simulink model "CCMSysServ"  (R2022b) (continued)
== system CCMSysServ == (continued)
      | <ReqID>NULL</ReqID>
      | <InitValue>0</InitValue>
  CarCfg_CarCfg790 : Inport  Description=|  Port=580  OutDataTypeStr=Nr2  PortDimensions=1
      | Energy adjustable type
      | <to>UsgModMgr</to>
      | <typ>Internal</typ>
      | <ReqID>22092</ReqID>
      | <InitValue>0</InitValue>
  CarCfg_CarCfg793 : Inport  Description=|  Port=581  OutDataTypeStr=Nr2  PortDimensions=1
      | VEHICLE TYPE
      | <to>All</to>
      | <typ>Internal</typ>
      | <ReqID>NULL</ReqID>
      | <InitValue>0</InitValue>
  CarCfg_CarCfg794 : Inport  Description=|  Port=582  OutDataTypeStr=Nr2  PortDimensions=1
      | FOOT SUPPORT
      | <to>All</to>
      | <typ>Internal</typ>
      | <ReqID>198565</ReqID>
      | <InitValue>0</InitValue>
  CarCfg_CarCfg798 : Inport  Description=|  Port=583  OutDataTypeStr=Nr2  PortDimensions=1
      | VEHICLE TYPE
      | <to>All</to>
      | <typ>Internal</typ>
      | <ReqID>NULL</ReqID>
      | <InitValue>0</InitValue>
  CarCfg_CarCfg8 : Inport  Description=|  Port=584  OutDataTypeStr=Nr2  PortDimensions=1
      | STEERING WHEEL POSITION
      | <to>All</to>
      | <typ>Internal</typ>
      | <ReqID>NULL</ReqID>
      | <InitValue>0</InitValue>
  CarCfg_CarCfg80 : Inport  Description=|  Port=585  OutDataTypeStr=Nr2  PortDimensions=1
      | KEYLESS ANTENNAS
      | <to>All</to>
      | <typ>Internal</typ>
      | <ReqID>NULL</ReqID>
      | <InitValue>0</InitValue>
  CarCfg_CarCfg81 : Inport  Description=|  Port=586  OutDataTypeStr=Nr2  PortDimensions=1
      | DOOR HANDLE TYPE
      | <to>All</to>
      | <typ>Internal</typ>
      | <ReqID>NULL</ReqID>
      | <InitValue>0</InitValue>
  CarCfg_CarCfg811 : Inport  Description=|  Port=587  OutDataTypeStr=Nr2  PortDimensions=1
      | VEHICLE TYPE
      | <to>All</to>
      | <typ>Internal</typ>
      | <ReqID>NULL</ReqID>
      | <InitValue>0</InitValue>
  CarCfg_CarCfg814 : Inport  Description=|  Port=588  OutDataTypeStr=Nr2  PortDimensions=1
      | VEHICLE TYPE
      | <to>All</to>
      | <typ>Internal</typ>
      | <ReqID>NULL</ReqID>
      | <InitValue>0</InitValue>
  CarCfg_CarCfg816 : Inport  Description=|  Port=589  OutDataTypeStr=Nr2  PortDimensions=1
      | VEHICLE TYPE
      | <to>All</to>
      | <typ>Internal</typ>
      | <ReqID>NULL</ReqID>
      | <InitValue>0</InitValue>
  CarCfg_CarCfg82 : Inport  Description=|  Port=590  OutDataTypeStr=Nr2  PortDimensions=1
      | CHARGE LID LOCK
      | <to>All</to>
      | <typ>Internal</typ>
      | <ReqID>NULL</ReqID>
      | <InitValue>0</InitValue>
  CarCfg_CarCfg822 : Inport  Description=|  Port=591  OutDataTypeStr=Nr2  PortDimensions=1
      | VEHICLE TYPE
      | <to>All</to>
      | <typ>Internal</typ>
      | <ReqID>NULL</ReqID>
      | <InitValue>0</InitValue>
  CarCfg_CarCfg826 : Inport  Description=|  Port=592  OutDataTypeStr=Nr2  PortDimensions=1
      | 12V Delayed Power Off
      | <to>All</to>
      | <typ>Internal</typ>
      | <ReqID>22010</ReqID>
      | <InitValue>0</InitValue>
  CarCfg_CarCfg829 : Inport  Description=|  Port=593  OutDataTypeStr=Nr2  PortDimensions=1
      | VEHICLE TYPE
      | <to>All</to>
      | <typ>Internal</typ>
      | <ReqID>NULL</ReqID>
      | <InitValue>0</InitValue>
  CarCfg_CarCfg83 : Inport  Description=|  Port=594  OutDataTypeStr=Nr2  PortDimensions=1
      | FUEL LID LOCK
      | <to>All</to>
      | <typ>Internal</typ>
      | <ReqID>NULL</ReqID>
      | <InitValue>0</InitValue>
  CarCfg_CarCfg831 : Inport  Description=|  Port=595  OutDataTypeStr=Nr2  PortDimensions=1
      | VEHICLE TYPE
      | <to>All</to>
      | <typ>Internal</typ>
      | <ReqID>NULL</ReqID>
      | <InitValue>0</InitValue>
  CarCfg_CarCfg834 : Inport  Description=|  Port=596  OutDataTypeStr=Nr2  PortDimensions=1
      | A2 Front position lamp
      | <to>All</to>
      | <typ>Internal</typ>
      | <ReqID>670781</ReqID>
      | <InitValue>0</InitValue>
  CarCfg_CarCfg835 : Inport  Description=|  Port=597  OutDataTypeStr=Nr2  PortDimensions=1
      | ROOF DRIVE TYPE
      | <to>All</to>
      | <typ>Internal</typ>
      | <ReqID>/</ReqID>
      | <InitValue>0</InitValue>
  CarCfg_CarCfg837 : Inport  Description=|  Port=598  OutDataTypeStr=Nr2  PortDimensions=1
      | Mobile phone remote monitoring and call
      | <to>UsgModMgr</to>
      | <typ>Internal</typ>
      | <ReqID>22019</ReqID>
      | <InitValue>0</InitValue>
  CarCfg_CarCfg839 : Inport  Description=|  Port=599  OutDataTypeStr=Nr2  PortDimensions=1
      | Energy adjustable type
      | <to>UsgModMgr</to>
      | <typ>Internal</typ>
      | <ReqID>22092</ReqID>
      | <InitValue>0</InitValue>
  CarCfg_CarCfg84 : Inport  Description=|  Port=600  OutDataTypeStr=Nr2  PortDimensions=1
      | CAR LOCK TYPE
      | <to>All</to>
      | <typ>Internal</typ>
      | <ReqID>NULL</ReqID>
      | <InitValue>0</InitValue>
  CarCfg_CarCfg841 : Inport  Description=|  Port=601  OutDataTypeStr=Nr2  PortDimensions=1
      | Electric lifting module
      | <to>All</to>
      | <typ>Internal</typ>
      | <ReqID>/</ReqID>
      | <InitValue>0</InitValue>
  CarCfg_CarCfg842 : Inport  Description=|  Port=602  OutDataTypeStr=Nr2  PortDimensions=1
      | Cabin oxygen supply system
      | <to>CCM</to>
      | <typ>Internal_other</typ>
      | <ReqID>871504</ReqID>
      | <InitValue>0</InitValue>
  CarCfg_CarCfg846 : Inport  Description=|  Port=603  OutDataTypeStr=Nr2  PortDimensions=1
      | RF KEY
      | <to>All</to>
      | <typ>Internal</typ>
      | <ReqID>/</ReqID>
      | <InitValue>0</InitValue>
  CarCfg_CarCfg848 : Inport  Description=|  Port=604  OutDataTypeStr=Nr2  PortDimensions=1
      | VEHICLE TYPE
      | <to>All</to>
      | <typ>Internal</typ>
      | <ReqID>NULL</ReqID>
      | <InitValue>0</InitValue>
  CarCfg_CarCfg849 : Inport  Port=605  OutDataTypeStr=Nr2  PortDimensions=1
  CarCfg_CarCfg85 : Inport  Description=|  Port=606  OutDataTypeStr=Nr2  PortDimensions=1
      | CENTRAL LOCK TYPE
      | <to>All</to>
      | <typ>Internal</typ>
      | <ReqID>NULL</ReqID>
      | <InitValue>0</InitValue>
  CarCfg_CarCfg851 : Inport  Description=|  Port=607  OutDataTypeStr=Nr2  PortDimensions=1
      | FLOATING DRVING
      | <to>CCM</to>
      | <typ>Internal_other</typ>
      | <ReqID>858088</ReqID>
      | <InitValue>0</InitValue>
  CarCfg_CarCfg855 : Inport  Description=|  Port=608  OutDataTypeStr=Nr2  PortDimensions=1
      | VEHICLE TYPE
      | <to>All</to>
      | <typ>Internal</typ>
      | <ReqID>NULL</ReqID>
      | <InitValue>0</InitValue>
  CarCfg_CarCfg857 : Inport  Description=|  Port=609  OutDataTypeStr=Nr2  PortDimensions=1
      | Ceiling Screen Control
      | <to>ALL</to>
      | <typ>Internal</typ>
      | <ReqID>/</ReqID>
      | <InitValue>0</InitValue>
  CarCfg_CarCfg858 : Inport  Description=|  Port=610  OutDataTypeStr=Nr2  PortDimensions=1
      | HIDDEN REAR WINDOW WIPER
      | <to>WiprWshrMgr</to>
      | <typ>Internal</typ>
      | <ReqID>169786</ReqID>
      | <InitValue>0</InitValue>
  CarCfg_CarCfg86 : Inport  Description=|  Port=611  OutDataTypeStr=Nr2  PortDimensions=1
      | CENTRAL LOCK SWITCHES
      | <to>All</to>
      | <typ>Internal</typ>
      | <ReqID>NULL</ReqID>
      | <InitValue>0</InitValue>
  CarCfg_CarCfg87 : Inport  Description=|  Port=612  OutDataTypeStr=Nr2  PortDimensions=1
      | CHILD LOCK, REAR DOORS
      | <to>All</to>
      | <typ>Internal</typ>
      | <ReqID>NULL</ReqID>
      | <InitValue>0</InitValue>
  CarCfg_CarCfg88 : Inport  Description=|  Port=613  OutDataTypeStr=Nr2  PortDimensions=1
      | PRIVATE LOCKING, LUGGAGE COMP.
      | <to>All</to>
      | <typ>Internal</typ>
      | <ReqID>NULL</ReqID>
      | <InitValue>0</InitValue>
  CarCfg_CarCfg89 : Inport  Description=|  Port=614  OutDataTypeStr=Nr2  PortDimensions=1
      | SLAM LOCKING
      | <to>All</to>
      | <typ>Internal</typ>
      | <ReqID>NULL</ReqID>
      | <InitValue>0</InitValue>
  CarCfg_CarCfg9 : Inport  Description=|  Port=615  OutDataTypeStr=Nr2  PortDimensions=1
      | GEARBOX
      | <to>All</to>
      | <typ>Internal</typ>
      | <ReqID>NULL</ReqID>
      | <InitValue>0</InitValue>
  CarCfg_CarCfg90 : Inport  Description=|  Port=616  OutDataTypeStr=Nr2  PortDimensions=1
      | AUTOMATIC LOCKING BY SPEED
      | <to>All</to>
      | <typ>Internal</typ>
      | <ReqID>NULL</ReqID>
      | <InitValue>0</InitValue>
  CarCfg_CarCfg91 : Inport  Description=|  Port=617  OutDataTypeStr=Nr2  PortDimensions=1
      | TWO STEP UNLOCKING
      | <to>All</to>
      | <typ>Internal</typ>
      | <ReqID>NULL</ReqID>
      | <InitValue>0</InitValue>
  CarCfg_CarCfg92 : Inport  Description=|  Port=618  OutDataTypeStr=Nr2  PortDimensions=1
      | FREQUENCY, REMOTE CONTROLS
      | <to>All</to>
      | <typ>Internal</typ>
      | <ReqID>NULL</ReqID>
      | <InitValue>0</InitValue>
  CarCfg_CarCfg93 : Inport  Description=|  Port=619  OutDataTypeStr=Nr2  PortDimensions=1
      | AUTHENTIC COMMUNICATION OF LOCKING COMMANDS
      | <to>All</to>
      | <typ>Internal</typ>
      | <ReqID>NULL</ReqID>
      | <InitValue>0</InitValue>
  CarCfg_CarCfg94 : Inport  Description=|  Port=620  OutDataTypeStr=Nr2  PortDimensions=1
      | KEYLESS ENTRY
      | <to>All</to>
      | <typ>Internal</typ>
      | <ReqID>NULL</ReqID>
      | <InitValue>0</InitValue>
  CarCfg_CarCfg95 : Inport  Description=|  Port=621  OutDataTypeStr=Nr2  PortDimensions=1
      | VISIBLE LOCKING/UNLOCKING
      | <to>All</to>
      | <typ>Internal</typ>
      | <ReqID>NULL</ReqID>
      | <InitValue>0</InitValue>
  CarCfg_CarCfg96 : Inport  Description=|  Port=622  OutDataTypeStr=Nr2  PortDimensions=1
      | AUDIBLE LOCKING FEEDBACK
      | <to>All</to>
      | <typ>Internal</typ>
      | <ReqID>NULL</ReqID>
      | <InitValue>0</InitValue>
  CarCfg_CarCfg97 : Inport  Description=|  Port=623  OutDataTypeStr=Nr2  PortDimensions=1
      | TRUNK RELEASE, POP-UP
      | <to>All</to>
      | <typ>Internal</typ>
      | <ReqID>NULL</ReqID>
      | <InitValue>0</InitValue>
  CarCfg_CarCfg98 : Inport  Description=|  Port=624  OutDataTypeStr=Nr2  PortDimensions=1
      | SELF OPENING BOOTLID/TAILGATE
      | <to>All</to>
      | <typ>Internal</typ>
      | <ReqID>NULL</ReqID>
      | <InitValue>0</InitValue>
  CarCfg_CarCfg99 : Inport  Description=|  Port=625  OutDataTypeStr=Nr2  PortDimensions=1
      | HANDSFREE OPENER, BOOTLID/TAILGATE
      | <to>All</to>
      | <typ>Internal</typ>
      | <ReqID>NULL</ReqID>
      | <InitValue>0</InitValue>
  VehCfgPrm_VehCfgPrm : Inport  Port=626  OutDataTypeStr=Bus: VehCfgPrm  PortDimensions=1  BusOutputAsStruct=on
  VehCfgPrmExt_VehCfgPrmExt : Inport  Port=627  OutDataTypeStr=Bus: VehCfgPrm  PortDimensions=1  BusOutputAsStruct=on
  VehModMngtGlbSafe1_VehModMngtGlbSafe1 : Inport  Port=628  OutDataTypeStr=Bus: VehModMngtGlbSafe1  PortDimensions=1  BusOutputAsStruct=on
  Vin_Vin : Inport  Port=629  OutDataTypeStr=Bus: Vin1  PortDimensions=1  BusOutputAsStruct=on
  BswLifeCycle_LifeCycle : Inport  Port=630  OutDataTypeStr=Enum: CCMLifeCycleModeGroup  PortDimensions=1
  CCMSysServ_Init : Subsystem
  CCMSysServ_Runnable_sys : Subsystem
  CCMSysServ_VehCfgPrmExt_sys : Subsystem
  CCMSysServ_VehCfgPrm_sys : Subsystem
  CCMSysServ_VehModMngtGlbSafe1_sys : Subsystem
  CCMSysServ_Vin_sys : Subsystem
  NvMBlockCarCfgData : DataStoreMemory  DataStoreName=NvMBlockCarCfgData  InitialValue=NvMBlockCarCfgDataInit  ShowAdditionalParam=off  Dimensions=62  StateMustResolveToSignalObject=on
  NvMBlockDidDataD005 : DataStoreMemory  DataStoreName=NvMBlockDidDataD005  InitialValue=NvMBlockDidDataD005Init  ShowAdditionalParam=off  Dimensions=90  StateMustResolveToSignalObject=on
  NvMBlockDidDataD09A : DataStoreMemory  DataStoreName=NvMBlockDidDataD09A  InitialValue=NvMBlockDidDataD09AInit  ShowAdditionalParam=off  Dimensions=1  StateMustResolveToSignalObject=on
  NvMBlockDidDataE103 : DataStoreMemory  DataStoreName=NvMBlockDidDataE103  InitialValue=NvMBlockDidDataE103Init  ShowAdditionalParam=off  Dimensions=70  StateMustResolveToSignalObject=on
  NvMBlockDidDataF190 : DataStoreMemory  DataStoreName=NvMBlockDidDataF190  InitialValue=NvMBlockDidDataF190Init  ShowAdditionalParam=off  Dimensions=17  StateMustResolveToSignalObject=on
  NvMBlockDidDataF1AB : DataStoreMemory  DataStoreName=NvMBlockDidDataF1AB  InitialValue=NvMBlockDidDataF1ABInit  ShowAdditionalParam=off  Dimensions=8  StateMustResolveToSignalObject=on
  SysServDtcService : Subsystem
  SysServParaService : Subsystem
  CarCfgForClimaCtrl_CarCfgFor3RDROWSEATHEAT : Outport  OutDataTypeStr=UInt8  PortDimensions=1
  CarCfgForClimaCtrl_CarCfgFor3RDROWSEATVENTILATED : Outport  Port=2  OutDataTypeStr=UInt8  PortDimensions=1
  CarCfgForClimaCtrl_CarCfgForBRAND : Outport  Port=3  OutDataTypeStr=UInt8  PortDimensions=1
  CarCfgForClimaCtrl_CarCfgForCabinOxgenSupply : Outport  Port=4  OutDataTypeStr=UInt8  PortDimensions=1
  CarCfgForClimaCtrl_CarCfgForElectricLiftingModule : Outport  Port=5  OutDataTypeStr=UInt8  PortDimensions=1
  CarCfgForClimaCtrl_CarCfgForExtVentnClimaCtrl : Outport  Port=6  OutDataTypeStr=Enum: CarCfgForExtVentnClimaCtrl  PortDimensions=1
  CarCfgForClimaCtrl_CarCfgForTEMPEREDSTORAGEBOX : Outport  Port=7  OutDataTypeStr=UInt8  PortDimensions=1
  CarCfgForClimaCtrl_CarCfgForFloatingDriving : Outport  Port=8  OutDataTypeStr=UInt8  PortDimensions=1
  CarCfgForClimaCtrl_CarCfgForInteriorViewCamera : Outport  Port=9  OutDataTypeStr=Enum: CarCfgForInteriorViewCamera  PortDimensions=1
  CarCfgForClimaCtrl_CarCfgForLifeDetection : Outport  Port=10  OutDataTypeStr=Enum: LifeDetection  PortDimensions=1
  CarCfgForClimaCtrl_CarCfgForMARKET : Outport  Port=11  OutDataTypeStr=UInt8  PortDimensions=1
  CarCfgForClimaCtrl_CarCfgForPowerSeats : Outport  Port=12  OutDataTypeStr=Enum: CarCfgForPowerSeats  PortDimensions=1
  CarCfgForClimaCtrl_CarCfgForPTCInFrontHVAC : Outport  Port=13  OutDataTypeStr=UInt8  PortDimensions=1
  CarCfgForClimaCtrl_CarCfgForRFKEY : Outport  Port=14  OutDataTypeStr=UInt8  PortDimensions=1
  CarCfgForClimaCtrl_CarCfgForVentLiftMotorExiSts : Outport  Port=15  OutDataTypeStr=UInt32  PortDimensions=1
  CarCfgForClimaCtrl_CarCfgForWadingAssistSystem : Outport  Port=16  OutDataTypeStr=UInt8  PortDimensions=1
  CarCfgForClimaCtrl_CarCfgWindFollowHumanMov : Outport  Port=17  OutDataTypeStr=UInt8  PortDimensions=1
  HvacCfgForClimaCtrl_HvacCfgForAQSTypes : Outport  Port=18  OutDataTypeStr=UInt8  PortDimensions=1
  HvacCfgForClimaCtrl_HvacCfgForHvacLowSideRETXVESts : Outport  Port=19  OutDataTypeStr=UInt8  PortDimensions=1
  HvacCfgForClimaCtrl_HvacCfgForHvacReBlowerType : Outport  Port=20  OutDataTypeStr=UInt8  PortDimensions=1
  HvacCfgForClimaCtrl_HvacCfgForHvacReVentPstn : Outport  Port=21  OutDataTypeStr=UInt8  PortDimensions=1
  HvacCfgForClimaCtrl_HvacCfgForHvacSnsrRCmptmtESts : Outport  Port=22  OutDataTypeStr=UInt8  PortDimensions=1
  HvacCfgForClimaCtrl_HvacCfgForHvacSnsrReHexESts : Outport  Port=23  OutDataTypeStr=UInt8  PortDimensions=1
  HvacCfgReqVersionReplace_HvacCfgReqVersionReplace : Outport  Port=24  OutDataTypeStr=UInt8  PortDimensions=1
  SysServCarCfgSts_SysServCCPLin2AQSSts : Outport  Port=25  OutDataTypeStr=Boolean  PortDimensions=1
  SysServCarCfgSts_SysServDUBlowerPwmSignTypeSts : Outport  Port=26  OutDataTypeStr=UInt8  PortDimensions=1
  SysServCarCfgSts_SysServDULin4HFAFStsH : Outport  Port=27  OutDataTypeStr=UInt32  PortDimensions=1
  SysServCarCfgSts_SysServDUReBlowerPwmSignSts : Outport  Port=28  OutDataTypeStr=UInt8  PortDimensions=1
  SysServCarCfgSts_SysServDUReBlowerPwmSts : Outport  Port=29  OutDataTypeStr=UInt8  PortDimensions=1
  SysServCarCfgSts_SysServFloatingMotorSts : Outport  Port=30  OutDataTypeStr=UInt8  PortDimensions=1
  SysServCarCfgSts_SysServFrntHEXSnsrPosn : Outport  Port=31  OutDataTypeStr=UInt8  PortDimensions=1
  SysServCarCfgSts_SysServLowSideRETXVSts : Outport  Port=32  OutDataTypeStr=UInt8  PortDimensions=1
  SysServCarCfgSts_SysServReHEXSnsrPosn : Outport  Port=33  OutDataTypeStr=UInt8  PortDimensions=1
  SysServCarCfgSts_SysServRHFASts : Outport  Port=34  OutDataTypeStr=UInt8  PortDimensions=1
  SysServCarCfgSts_SysServSnsrForFCmptmtTType : Outport  Port=35  OutDataTypeStr=UInt8  PortDimensions=1
  CarCfgForClimaCtrl_CarCfgForAutDemst : Outport  Port=36  OutDataTypeStr=UInt8  PortDimensions=1
  CarCfgForClimaCtrl_CarCfgForAirClng : Outport  Port=37  OutDataTypeStr=Enum: CarCfgForAirClng  PortDimensions=1
  CarCfgForClimaCtrl_CarCfgForAirClnrAdv : Outport  Port=38  OutDataTypeStr=UInt8  PortDimensions=1
  CarCfgForClimaCtrl_CarCfgForAirFrag : Outport  Port=39  OutDataTypeStr=Enum: CarCfgForAirFrag  PortDimensions=1
  CarCfgForClimaCtrl_CarCfgForAirMtrlSnsr : Outport  Port=40  OutDataTypeStr=Enum: CarCfgForAirMtrlSnsrGly  PortDimensions=1
  CarCfgForClimaCtrl_CarCfgForAirQly : Outport  Port=41  OutDataTypeStr=Enum: CarCfgForAirQlyGly  PortDimensions=1
  CarCfgForClimaCtrl_CarCfgForAmntOfSeat : Outport  Port=42  OutDataTypeStr=Enum: CarCfgForAmntOfSeatTyp3  PortDimensions=1
  CarCfgForClimaCtrl_CarCfgForAuxWtrPmp : Outport  Port=43  OutDataTypeStr=Enum: CarCfgForAuxWtrPmp  PortDimensions=1
  CarCfgForClimaCtrl_CarCfgForBypVlvInHvac : Outport  Port=44  OutDataTypeStr=Enum: CarCfgForBypVlvInHvac2  PortDimensions=1
  CarCfgForClimaCtrl_CarCfgForClsVlvInHvac : Outport  Port=45  OutDataTypeStr=Enum: CarCfgForClsVlvInHvac  PortDimensions=1
  CarCfgForClimaCtrl_CarCfgForCmprAcTyp : Outport  Port=46  OutDataTypeStr=UInt8  PortDimensions=1
  CarCfgForClimaCtrl_CarCfgForCmptmtClng : Outport  Port=47  OutDataTypeStr=Enum: CarCfgForCmptmtClng  PortDimensions=1
  CarCfgForClimaCtrl_CarCfgForElecVentAdjmt : Outport  Port=48  OutDataTypeStr=Enum: CarCfgForElecVentAdjmt  PortDimensions=1
  CarCfgForClimaCtrl_CarCfgForFlcTyp : Outport  Port=49  OutDataTypeStr=Enum: CarCfgForFlcTyp  PortDimensions=1
  CarCfgForClimaCtrl_CarCfgForFoldCSD : Outport  Port=50  OutDataTypeStr=Enum: CarCfgForFoldCSD  PortDimensions=1
  CarCfgForClimaCtrl_CarCfgForFuTyp : Outport  Port=51  OutDataTypeStr=Enum: CarCfgForFuTyp  PortDimensions=1
  CarCfgForClimaCtrl_CarCfgForGlassTyp : Outport  Port=52  OutDataTypeStr=Enum: CarCfgForGlassTyp  PortDimensions=1
  CarCfgForClimaCtrl_CarCfgForHeadRadar : Outport  Port=53  OutDataTypeStr=Enum: HeadRadar  PortDimensions=1
  CarCfgForClimaCtrl_CarCfgForHeatPmp : Outport  Port=54  OutDataTypeStr=Enum: CarCfgForHeatPmpTyp  PortDimensions=1
  CarCfgForClimaCtrl_CarCfgForHeatrDurgDrvg : Outport  Port=55  OutDataTypeStr=Enum: CarCfgForHeatrDurgDrvg  PortDimensions=1
  CarCfgForClimaCtrl_CarCfgForHeatrTyp : Outport  Port=56  OutDataTypeStr=UInt8  PortDimensions=1
  CarCfgForClimaCtrl_CarCfgForHvacFilTyp : Outport  Port=57  OutDataTypeStr=Enum: CarCfgForHvacFilTypGly  PortDimensions=1
  CarCfgForClimaCtrl_CarCfgForHvacFlrSnsrForT : Outport  Port=58  OutDataTypeStr=Enum: CarCfgForHvacFlrSnsrForT  PortDimensions=1
  CarCfgForClimaCtrl_CarCfgForHvacHeatrSnsrT : Outport  Port=59  OutDataTypeStr=Enum: CarCfgForHvacHeatrSnsrT  PortDimensions=1
  CarCfgForClimaCtrl_CarCfgForHvacRe : Outport  Port=60  OutDataTypeStr=UInt8  PortDimensions=1
  CarCfgForClimaCtrl_CarCfgForHvacTyp : Outport  Port=61  OutDataTypeStr=UInt8  PortDimensions=1
  CarCfgForClimaCtrl_CarCfgForLoVoltElecCfg : Outport  Port=62  OutDataTypeStr=Enum: CarCfgForLoVoltElecCfg  PortDimensions=1
  CarCfgForClimaCtrl_CarCfgForNavSysandIntnmap : Outport  Port=63  OutDataTypeStr=Enum: CarCfgForNavSysandIntnmap  PortDimensions=1
  CarCfgForClimaCtrl_CarCfgForNrOfDoor : Outport  Port=64  OutDataTypeStr=Enum: CarCfgForNrOfDoor2  PortDimensions=1
  CarCfgForClimaCtrl_CarCfgForPipeInFuTank : Outport  Port=65  OutDataTypeStr=Enum: CarCfgForPipeInFuTank  PortDimensions=1
  CarCfgForClimaCtrl_CarCfgForPreClng : Outport  Port=66  OutDataTypeStr=Enum: CarCfgForPreClng  PortDimensions=1
  CarCfgForClimaCtrl_CarCfgForPrkgCDSNotAvl : Outport  Port=67  OutDataTypeStr=Enum: CarCfgForPrkgCDSNotAvl  PortDimensions=1
  CarCfgForClimaCtrl_CarCfgForPrkgClima : Outport  Port=68  OutDataTypeStr=Enum: CarCfgForPrkgClima  PortDimensions=1
  CarCfgForClimaCtrl_CarCfgForPrpsnTyp : Outport  Port=69  OutDataTypeStr=Enum: CarCfgForPrpsnTypGly  PortDimensions=1
  CarCfgForClimaCtrl_CarCfgForRemEngStrtPreCdn : Outport  Port=70  OutDataTypeStr=Enum: CarCfgForRemEngStrtPreCdn  PortDimensions=1
  CarCfgForClimaCtrl_CarCfgForRoofHatch : Outport  Port=71  OutDataTypeStr=Enum: CarCfgForRoofHatch  PortDimensions=1
  CarCfgForClimaCtrl_CarCfgForSeatHeatgCal : Outport  Port=72  OutDataTypeStr=Enum: CarCfgForSeatHeatgCal1  PortDimensions=1
  CarCfgForClimaCtrl_CarCfgForSeatHeatgForRowFirst : Outport  Port=73  OutDataTypeStr=Enum: CarCfgForSeatHeatgForRowFirst  PortDimensions=1
  CarCfgForClimaCtrl_CarCfgForSeatHeatgForRowSec : Outport  Port=74  OutDataTypeStr=Enum: CarCfgForSeatHeatgForRowSec  PortDimensions=1
  CarCfgForClimaCtrl_CarCfgForSeatVentnForRowFirst : Outport  Port=75  OutDataTypeStr=Enum: CarCfgForSeatVentnForRowFirst1  PortDimensions=1
  CarCfgForClimaCtrl_CarCfgForSeatVentnForRowSec : Outport  Port=76  OutDataTypeStr=Enum: CarCfgForSeatVentnForRowSecGly  PortDimensions=1
  CarCfgForClimaCtrl_CarCfgForSecRowVentnCenter : Outport  Port=77  OutDataTypeStr=Enum: CarCfgForSecRowVentnCenter  PortDimensions=1
  CarCfgForClimaCtrl_CarCfgForSecRowVentnSide : Outport  Port=78  OutDataTypeStr=Enum: CarCfgForSecRowVentnSide  PortDimensions=1
  CarCfgForClimaCtrl_CarCfgForSmallFlowCyc : Outport  Port=79  OutDataTypeStr=Enum: CarCfgForSmallFlowCyc  PortDimensions=1
  CarCfgForClimaCtrl_CarCfgForSmkSnsr : Outport  Port=80  OutDataTypeStr=Enum: CarCfgForSmkSnsr  PortDimensions=1
  CarCfgForClimaCtrl_CarCfgForSnsrForCmptmtT : Outport  Port=81  OutDataTypeStr=Enum: CarCfgForSnsrForCmptmtT  PortDimensions=1
  CarCfgForClimaCtrl_CarCfgForSnsrHumPrsnt : Outport  Port=82  OutDataTypeStr=Enum: CarCfgForSnsrHumPrsnt  PortDimensions=1
  CarCfgForClimaCtrl_CarCfgForStartDevice : Outport  Port=83  OutDataTypeStr=Enum: StartDevice  PortDimensions=1
  CarCfgForClimaCtrl_CarCfgForSteerWhlHeatg : Outport  Port=84  OutDataTypeStr=UInt8  PortDimensions=1
  CarCfgForClimaCtrl_CarCfgForSteerWhlPosn : Outport  Port=85  OutDataTypeStr=Enum: CarCfgForSteerWhlPosn  PortDimensions=1
  CarCfgForClimaCtrl_CarCfgForSunSnsr : Outport  Port=86  OutDataTypeStr=Enum: CarCfgForSunSnsr  PortDimensions=1
  CarCfgForClimaCtrl_CarCfgForTelmNodTyp : Outport  Port=87  OutDataTypeStr=Enum: CarCfgForTelmNodTyp  PortDimensions=1
  CarCfgForClimaCtrl_CarCfgForThermMngt : Outport  Port=88  OutDataTypeStr=Enum: CarCfgForThermMngt  PortDimensions=1
  CarCfgForClimaCtrl_CarCfgForVehTyp : Outport  Port=89  OutDataTypeStr=Enum: CarCfgForVehTyp3  PortDimensions=1
  CarCfgForClimaCtrl_CarCfgForVgaMotorExistSts : Outport  Port=90  OutDataTypeStr=CarCfgForVgaMotorExistSts  PortDimensions=1
  CarCfgForClimaCtrl_CarCfgForWindHeatd : Outport  Port=91  OutDataTypeStr=Enum: CarCfgForWindHeatd  PortDimensions=1
  HvacCfgForClimaCtrl_HvacCfgForHvacFrontBlowerType : Outport  Port=92  OutDataTypeStr=HvacCfgForHvacFrontBlowerType  PortDimensions=1
  HvacCfgForClimaCtrl_HvacCfgForHvacMiscExistSts : Outport  Port=93  OutDataTypeStr=HvacCfgForHvacMiscExistSts  PortDimensions=1
  HvacCfgForClimaCtrl_HvacCfgForHvacMotorExistSts : Outport  Port=94  OutDataTypeStr=HvacCfgForHvacMotorExistSts  PortDimensions=1
  HvacCfgForClimaCtrl_HvacCfgForHvacSensorExistSts : Outport  Port=95  OutDataTypeStr=HvacCfgForHvacSensorExistSts  PortDimensions=1
  SysServCarCfgSts_SysServCarID : Outport  Port=96  OutDataTypeStr=Enum: SysServCarID  PortDimensions=1
  SysServCarCfgSts_SysServCCPAQSSts : Outport  Port=97  OutDataTypeStr=UInt8  PortDimensions=1
  SysServCarCfgSts_SysServCCPBGMNum : Outport  Port=98  OutDataTypeStr=UInt8  PortDimensions=1
  SysServCarCfgSts_SysServCCPHEXSensorSts : Outport  Port=99  OutDataTypeStr=UInt8  PortDimensions=1
  SysServCarCfgSts_SysServCCPLin2AFUSts : Outport  Port=100  OutDataTypeStr=Boolean  PortDimensions=1
  SysServCarCfgSts_SysServCCPLin2AIUSts : Outport  Port=101  OutDataTypeStr=UInt8  PortDimensions=1
  SysServCarCfgSts_SysServCCPLin2CDSSts : Outport  Port=102  OutDataTypeStr=Boolean  PortDimensions=1
  SysServCarCfgSts_SysServCCPLin2PMSISts : Outport  Port=103  OutDataTypeStr=Boolean  PortDimensions=1
  SysServCarCfgSts_SysServCCPLin3VGASts : Outport  Port=104  OutDataTypeStr=Boolean  PortDimensions=1
  SysServCarCfgSts_SysServDUAirVentSnrSts : Outport  Port=105  OutDataTypeStr=Boolean  PortDimensions=1
  SysServCarCfgSts_SysServDUBlowerPwmSignSts : Outport  Port=106  OutDataTypeStr=Boolean  PortDimensions=1
  SysServCarCfgSts_SysServDUBlowerPwmSts : Outport  Port=107  OutDataTypeStr=Boolean  PortDimensions=1
  SysServCarCfgSts_SysServDUDCDefrostSts : Outport  Port=108  OutDataTypeStr=UInt8  PortDimensions=1
  SysServCarCfgSts_SysServDUDCFLMdeSts : Outport  Port=109  OutDataTypeStr=UInt8  PortDimensions=1
  SysServCarCfgSts_SysServDUDCModeSts : Outport  Port=110  OutDataTypeStr=UInt8  PortDimensions=1
  SysServCarCfgSts_SysServDUDCRecSts : Outport  Port=111  OutDataTypeStr=UInt8  PortDimensions=1
  SysServCarCfgSts_SysServDUDCSModeSts : Outport  Port=112  OutDataTypeStr=UInt8  PortDimensions=1
  SysServCarCfgSts_SysServDUDCSTLSts : Outport  Port=113  OutDataTypeStr=UInt8  PortDimensions=1
  SysServCarCfgSts_SysServDUDCTLSts : Outport  Port=114  OutDataTypeStr=UInt8  PortDimensions=1
  SysServCarCfgSts_SysServDUDCTRSts : Outport  Port=115  OutDataTypeStr=UInt8  PortDimensions=1
  SysServCarCfgSts_SysServDUFLFootSts : Outport  Port=116  OutDataTypeStr=UInt8  PortDimensions=1
  SysServCarCfgSts_SysServDUFLVentSts : Outport  Port=117  OutDataTypeStr=UInt8  PortDimensions=1
  SysServCarCfgSts_SysServDUFRFootSts : Outport  Port=118  OutDataTypeStr=UInt8  PortDimensions=1
  SysServCarCfgSts_SysServDUFRVentSts : Outport  Port=119  OutDataTypeStr=UInt8  PortDimensions=1
  SysServCarCfgSts_SysServDUIncarMotorSts : Outport  Port=120  OutDataTypeStr=Boolean  PortDimensions=1
  SysServCarCfgSts_SysServDULin1HBMFSts : Outport  Port=121  OutDataTypeStr=Boolean  PortDimensions=1
  SysServCarCfgSts_SysServDULin4HFAFSts : Outport  Port=122  OutDataTypeStr=UInt32  PortDimensions=1
  SysServCarCfgSts_SysServDUSFLFootSts : Outport  Port=123  OutDataTypeStr=UInt8  PortDimensions=1
  SysServCarCfgSts_SysServDUSFLVentSts : Outport  Port=124  OutDataTypeStr=UInt8  PortDimensions=1
  SysServCarCfgSts_SysServDUSFRFootSts : Outport  Port=125  OutDataTypeStr=UInt8  PortDimensions=1
  SysServCarCfgSts_SysServDUSFRVentSts : Outport  Port=126  OutDataTypeStr=UInt8  PortDimensions=1
  SysServCarCfgSts_SysServDUSnsrForFCmptmtTSts : Outport  Port=127  OutDataTypeStr=UInt8  PortDimensions=1
  SysServCarCfgSts_SysServDUSnsrForRair : Outport  Port=128  OutDataTypeStr=UInt8  PortDimensions=1
  SysServCarCfgSts_SysServDUSnsrForRCmptmtTSts : Outport  Port=129  OutDataTypeStr=UInt8  PortDimensions=1
  SysServEcuSlpDelayFinish_EcuSlpDelayFinishFlg : Outport  Port=130  OutDataTypeStr=UInt8  PortDimensions=1
  dp_LifeCycle_LifeCycle : Outport  Port=131  OutDataTypeStr=Enum: CCMLifeCycleModeGroup  PortDimensions=1
  wires:
  ComMPNCState_PNCState/1 -> CCMSysServ_Runnable_sys/1(ComMPNCState_PNCState_read)
  ComMPNCState_VFCState/1 -> CCMSysServ_Runnable_sys/2(ComMPNCState_VFCState_read)
  EcuKeepActv_EcuKeepActv/1 -> CCMSysServ_Runnable_sys/3(EcuKeepActv_EcuKeepActv_read)
  CarTiGlb_CarTiGlb/1 -> CCMSysServ_Runnable_sys/4(CarTiGlb_CarTiGlb_read)
  CarTiGlb_CarTiGlb_IsUpdated/1 -> CCMSysServ_Runnable_sys/5(CarTiGlb_CarTiGlb_IsUpdated_read)
  ActrFrntReqForRowFirstLeModDistbn_ActrFrntReqForRowFirstLeModDistbn/1 -> CCMSysServ_Runnable_sys/6(ActrFrntReqForRowFirstLeModDistbn_ActrFrntReqForRowFirstLeModDistbn_read)
  CarCfg_CarCfg1/1 -> CCMSysServ_Runnable_sys/7(CarCfg_CarCfg1_read)
  CarCfg_CarCfg10/1 -> CCMSysServ_Runnable_sys/8(CarCfg_CarCfg10_read)
  CarCfg_CarCfg100/1 -> CCMSysServ_Runnable_sys/9(CarCfg_CarCfg100_read)
  CarCfg_CarCfg1002/1 -> CCMSysServ_Runnable_sys/10(CarCfg_CarCfg1002_read)
  CarCfg_CarCfg1004/1 -> CCMSysServ_Runnable_sys/11(CarCfg_CarCfg1004_read)
  CarCfg_CarCfg1007/1 -> CCMSysServ_Runnable_sys/12(CarCfg_CarCfg1007_read)
  CarCfg_CarCfg101/1 -> CCMSysServ_Runnable_sys/13(CarCfg_CarCfg101_read)
  CarCfg_CarCfg1011/1 -> CCMSysServ_Runnable_sys/14(CarCfg_CarCfg1011_read)
  CarCfg_CarCfg1017/1 -> CCMSysServ_Runnable_sys/15(CarCfg_CarCfg1017_read)
  CarCfg_CarCfg1018/1 -> CCMSysServ_Runnable_sys/16(CarCfg_CarCfg1018_read)
  CarCfg_CarCfg102/1 -> CCMSysServ_Runnable_sys/17(CarCfg_CarCfg102_read)
  CarCfg_CarCfg103/1 -> CCMSysServ_Runnable_sys/18(CarCfg_CarCfg103_read)
  CarCfg_CarCfg1034/1 -> CCMSysServ_Runnable_sys/19(CarCfg_CarCfg1034_read)
  CarCfg_CarCfg1038/1 -> CCMSysServ_Runnable_sys/20(CarCfg_CarCfg1038_read)
  CarCfg_CarCfg1039/1 -> CCMSysServ_Runnable_sys/21(CarCfg_CarCfg1039_read)
  CarCfg_CarCfg104/1 -> CCMSysServ_Runnable_sys/22(CarCfg_CarCfg104_read)
  CarCfg_CarCfg1042/1 -> CCMSysServ_Runnable_sys/23(CarCfg_CarCfg1042_read)
  CarCfg_CarCfg1043/1 -> CCMSysServ_Runnable_sys/24(CarCfg_CarCfg1043_read)
  CarCfg_CarCfg1044/1 -> CCMSysServ_Runnable_sys/25(CarCfg_CarCfg1044_read)
  CarCfg_CarCfg1045/1 -> CCMSysServ_Runnable_sys/26(CarCfg_CarCfg1045_read)
  CarCfg_CarCfg1047/1 -> CCMSysServ_Runnable_sys/27(CarCfg_CarCfg1047_read)
  CarCfg_CarCfg105/1 -> CCMSysServ_Runnable_sys/28(CarCfg_CarCfg105_read)
  CarCfg_CarCfg1052/1 -> CCMSysServ_Runnable_sys/29(CarCfg_CarCfg1052_read)
  CarCfg_CarCfg1057/1 -> CCMSysServ_Runnable_sys/30(CarCfg_CarCfg1057_read)
  CarCfg_CarCfg106/1 -> CCMSysServ_Runnable_sys/31(CarCfg_CarCfg106_read)
  CarCfg_CarCfg1061/1 -> CCMSysServ_Runnable_sys/32(CarCfg_CarCfg1061_read)
  CarCfg_CarCfg1063/1 -> CCMSysServ_Runnable_sys/33(CarCfg_CarCfg1063_read)
  CarCfg_CarCfg1065/1 -> CCMSysServ_Runnable_sys/34(CarCfg_CarCfg1065_read)
  CarCfg_CarCfg107/1 -> CCMSysServ_Runnable_sys/35(CarCfg_CarCfg107_read)
  CarCfg_CarCfg1075/1 -> CCMSysServ_Runnable_sys/36(CarCfg_CarCfg1075_read)
  CarCfg_CarCfg1077/1 -> CCMSysServ_Runnable_sys/37(CarCfg_CarCfg1077_read)
  CarCfg_CarCfg108/1 -> CCMSysServ_Runnable_sys/38(CarCfg_CarCfg108_read)
  CarCfg_CarCfg1080/1 -> CCMSysServ_Runnable_sys/39(CarCfg_CarCfg1080_read)
  CarCfg_CarCfg1083/1 -> CCMSysServ_Runnable_sys/40(CarCfg_CarCfg1083_read)
  CarCfg_CarCfg1087/1 -> CCMSysServ_Runnable_sys/41(CarCfg_CarCfg1087_read)
  CarCfg_CarCfg1088/1 -> CCMSysServ_Runnable_sys/42(CarCfg_CarCfg1088_read)
  CarCfg_CarCfg109/1 -> CCMSysServ_Runnable_sys/43(CarCfg_CarCfg109_read)
  CarCfg_CarCfg1090/1 -> CCMSysServ_Runnable_sys/44(CarCfg_CarCfg1090_read)
  CarCfg_CarCfg1096/1 -> CCMSysServ_Runnable_sys/45(CarCfg_CarCfg1096_read)
  CarCfg_CarCfg110/1 -> CCMSysServ_Runnable_sys/46(CarCfg_CarCfg110_read)
  CarCfg_CarCfg1101/1 -> CCMSysServ_Runnable_sys/47(CarCfg_CarCfg1101_read)
  CarCfg_CarCfg1102/1 -> CCMSysServ_Runnable_sys/48(CarCfg_CarCfg1102_read)
  CarCfg_CarCfg1103/1 -> CCMSysServ_Runnable_sys/49(CarCfg_CarCfg1103_read)
  CarCfg_CarCfg1104/1 -> CCMSysServ_Runnable_sys/50(CarCfg_CarCfg1104_read)
  CarCfg_CarCfg111/1 -> CCMSysServ_Runnable_sys/51(CarCfg_CarCfg111_read)
  CarCfg_CarCfg1111/1 -> CCMSysServ_Runnable_sys/52(CarCfg_CarCfg1111_read)
  CarCfg_CarCfg1112/1 -> CCMSysServ_Runnable_sys/53(CarCfg_CarCfg1112_read)
  CarCfg_CarCfg112/1 -> CCMSysServ_Runnable_sys/54(CarCfg_CarCfg112_read)
  CarCfg_CarCfg113/1 -> CCMSysServ_Runnable_sys/55(CarCfg_CarCfg113_read)
  CarCfg_CarCfg1130/1 -> CCMSysServ_Runnable_sys/56(CarCfg_CarCfg1130_read)
  CarCfg_CarCfg1134/1 -> CCMSysServ_Runnable_sys/57(CarCfg_CarCfg1134_read)
  CarCfg_CarCfg1136/1 -> CCMSysServ_Runnable_sys/58(CarCfg_CarCfg1136_read)
  CarCfg_CarCfg114/1 -> CCMSysServ_Runnable_sys/59(CarCfg_CarCfg114_read)
  CarCfg_CarCfg115/1 -> CCMSysServ_Runnable_sys/60(CarCfg_CarCfg115_read)
  CarCfg_CarCfg1154/1 -> CCMSysServ_Runnable_sys/61(CarCfg_CarCfg1154_read)
  CarCfg_CarCfg116/1 -> CCMSysServ_Runnable_sys/62(CarCfg_CarCfg116_read)
  CarCfg_CarCfg1166/1 -> CCMSysServ_Runnable_sys/63(CarCfg_CarCfg1166_read)
  CarCfg_CarCfg117/1 -> CCMSysServ_Runnable_sys/64(CarCfg_CarCfg117_read)
  CarCfg_CarCfg1170/1 -> CCMSysServ_Runnable_sys/65(CarCfg_CarCfg1170_read)
  CarCfg_CarCfg1171/1 -> CCMSysServ_Runnable_sys/66(CarCfg_CarCfg1171_read)
  CarCfg_CarCfg1172/1 -> CCMSysServ_Runnable_sys/67(CarCfg_CarCfg1172_read)
  CarCfg_CarCfg1173/1 -> CCMSysServ_Runnable_sys/68(CarCfg_CarCfg1173_read)
  CarCfg_CarCfg1174/1 -> CCMSysServ_Runnable_sys/69(CarCfg_CarCfg1174_read)
  CarCfg_CarCfg1175/1 -> CCMSysServ_Runnable_sys/70(CarCfg_CarCfg1175_read)
  CarCfg_CarCfg1177/1 -> CCMSysServ_Runnable_sys/71(CarCfg_CarCfg1177_read)
  CarCfg_CarCfg1178/1 -> CCMSysServ_Runnable_sys/72(CarCfg_CarCfg1178_read)
  CarCfg_CarCfg118/1 -> CCMSysServ_Runnable_sys/73(CarCfg_CarCfg118_read)
  CarCfg_CarCfg1184/1 -> CCMSysServ_Runnable_sys/74(CarCfg_CarCfg1184_read)
  CarCfg_CarCfg1185/1 -> CCMSysServ_Runnable_sys/75(CarCfg_CarCfg1185_read)
  CarCfg_CarCfg1189/1 -> CCMSysServ_Runnable_sys/76(CarCfg_CarCfg1189_read)
  CarCfg_CarCfg119/1 -> CCMSysServ_Runnable_sys/77(CarCfg_CarCfg119_read)
  CarCfg_CarCfg12/1 -> CCMSysServ_Runnable_sys/78(CarCfg_CarCfg12_read)
  CarCfg_CarCfg120/1 -> CCMSysServ_Runnable_sys/79(CarCfg_CarCfg120_read)
  CarCfg_CarCfg121/1 -> CCMSysServ_Runnable_sys/80(CarCfg_CarCfg121_read)
  CarCfg_CarCfg1214/1 -> CCMSysServ_Runnable_sys/81(CarCfg_CarCfg1214_read)
  CarCfg_CarCfg1216/1 -> CCMSysServ_Runnable_sys/82(CarCfg_CarCfg1216_read)
  CarCfg_CarCfg1217/1 -> CCMSysServ_Runnable_sys/83(CarCfg_CarCfg1217_read)
  CarCfg_CarCfg1218/1 -> CCMSysServ_Runnable_sys/84(CarCfg_CarCfg1218_read)
  CarCfg_CarCfg1219/1 -> CCMSysServ_Runnable_sys/85(CarCfg_CarCfg1219_read)
  CarCfg_CarCfg122/1 -> CCMSysServ_Runnable_sys/86(CarCfg_CarCfg122_read)
  CarCfg_CarCfg123/1 -> CCMSysServ_Runnable_sys/87(CarCfg_CarCfg123_read)
  CarCfg_CarCfg1230/1 -> CCMSysServ_Runnable_sys/88(CarCfg_CarCfg1230_read)
  CarCfg_CarCfg1231/1 -> CCMSysServ_Runnable_sys/89(CarCfg_CarCfg1231_read)
  CarCfg_CarCfg1232/1 -> CCMSysServ_Runnable_sys/90(CarCfg_CarCfg1232_read)
  CarCfg_CarCfg1233/1 -> CCMSysServ_Runnable_sys/91(CarCfg_CarCfg1233_read)
  CarCfg_CarCfg1234/1 -> CCMSysServ_Runnable_sys/92(CarCfg_CarCfg1234_read)
  CarCfg_CarCfg124/1 -> CCMSysServ_Runnable_sys/93(CarCfg_CarCfg124_read)
  CarCfg_CarCfg1247/1 -> CCMSysServ_Runnable_sys/94(CarCfg_CarCfg1247_read)
  CarCfg_CarCfg125/1 -> CCMSysServ_Runnable_sys/95(CarCfg_CarCfg125_read)
  CarCfg_CarCfg1250/1 -> CCMSysServ_Runnable_sys/96(CarCfg_CarCfg1250_read)
  CarCfg_CarCfg1251/1 -> CCMSysServ_Runnable_sys/97(CarCfg_CarCfg1251_read)
  CarCfg_CarCfg1258/1 -> CCMSysServ_Runnable_sys/98(CarCfg_CarCfg1258_read)
  CarCfg_CarCfg1259/1 -> CCMSysServ_Runnable_sys/99(CarCfg_CarCfg1259_read)
  CarCfg_CarCfg126/1 -> CCMSysServ_Runnable_sys/100(CarCfg_CarCfg126_read)
  CarCfg_CarCfg1260/1 -> CCMSysServ_Runnable_sys/101(CarCfg_CarCfg1260_read)
  CarCfg_CarCfg1261/1 -> CCMSysServ_Runnable_sys/102(CarCfg_CarCfg1261_read)
  CarCfg_CarCfg1264/1 -> CCMSysServ_Runnable_sys/103(CarCfg_CarCfg1264_read)
  CarCfg_CarCfg1266/1 -> CCMSysServ_Runnable_sys/104(CarCfg_CarCfg1266_read)
  CarCfg_CarCfg1267/1 -> CCMSysServ_Runnable_sys/105(CarCfg_CarCfg1267_read)
  CarCfg_CarCfg127/1 -> CCMSysServ_Runnable_sys/106(CarCfg_CarCfg127_read)
  CarCfg_CarCfg1273/1 -> CCMSysServ_Runnable_sys/107(CarCfg_CarCfg1273_read)
  CarCfg_CarCfg1275/1 -> CCMSysServ_Runnable_sys/108(CarCfg_CarCfg1275_read)
  CarCfg_CarCfg1277/1 -> CCMSysServ_Runnable_sys/109(CarCfg_CarCfg1277_read)
  CarCfg_CarCfg1278/1 -> CCMSysServ_Runnable_sys/110(CarCfg_CarCfg1278_read)
  CarCfg_CarCfg1279/1 -> CCMSysServ_Runnable_sys/111(CarCfg_CarCfg1279_read)
  CarCfg_CarCfg128/1 -> CCMSysServ_Runnable_sys/112(CarCfg_CarCfg128_read)
  CarCfg_CarCfg1283/1 -> CCMSysServ_Runnable_sys/113(CarCfg_CarCfg1283_read)
  CarCfg_CarCfg1285/1 -> CCMSysServ_Runnable_sys/114(CarCfg_CarCfg1285_read)
  CarCfg_CarCfg1286/1 -> CCMSysServ_Runnable_sys/115(CarCfg_CarCfg1286_read)
  CarCfg_CarCfg1287/1 -> CCMSysServ_Runnable_sys/116(CarCfg_CarCfg1287_read)
  CarCfg_CarCfg129/1 -> CCMSysServ_Runnable_sys/117(CarCfg_CarCfg129_read)
  CarCfg_CarCfg13/1 -> CCMSysServ_Runnable_sys/118(CarCfg_CarCfg13_read)
  CarCfg_CarCfg130/1 -> CCMSysServ_Runnable_sys/119(CarCfg_CarCfg130_read)
  CarCfg_CarCfg131/1 -> CCMSysServ_Runnable_sys/120(CarCfg_CarCfg131_read)
  CarCfg_CarCfg132/1 -> CCMSysServ_Runnable_sys/121(CarCfg_CarCfg132_read)
  CarCfg_CarCfg133/1 -> CCMSysServ_Runnable_sys/122(CarCfg_CarCfg133_read)
  CarCfg_CarCfg134/1 -> CCMSysServ_Runnable_sys/123(CarCfg_CarCfg134_read)
  CarCfg_CarCfg135/1 -> CCMSysServ_Runnable_sys/124(CarCfg_CarCfg135_read)
  CarCfg_CarCfg136/1 -> CCMSysServ_Runnable_sys/125(CarCfg_CarCfg136_read)
  CarCfg_CarCfg137/1 -> CCMSysServ_Runnable_sys/126(CarCfg_CarCfg137_read)
  CarCfg_CarCfg138/1 -> CCMSysServ_Runnable_sys/127(CarCfg_CarCfg138_read)
  CarCfg_CarCfg139/1 -> CCMSysServ_Runnable_sys/128(CarCfg_CarCfg139_read)
  CarCfg_CarCfg14/1 -> CCMSysServ_Runnable_sys/129(CarCfg_CarCfg14_read)
  CarCfg_CarCfg140/1 -> CCMSysServ_Runnable_sys/130(CarCfg_CarCfg140_read)
  CarCfg_CarCfg141/1 -> CCMSysServ_Runnable_sys/131(CarCfg_CarCfg141_read)
  CarCfg_CarCfg142/1 -> CCMSysServ_Runnable_sys/132(CarCfg_CarCfg142_read)
  CarCfg_CarCfg143/1 -> CCMSysServ_Runnable_sys/133(CarCfg_CarCfg143_read)
  CarCfg_CarCfg144/1 -> CCMSysServ_Runnable_sys/134(CarCfg_CarCfg144_read)
  CarCfg_CarCfg145/1 -> CCMSysServ_Runnable_sys/135(CarCfg_CarCfg145_read)
  CarCfg_CarCfg146/1 -> CCMSysServ_Runnable_sys/136(CarCfg_CarCfg146_read)
  CarCfg_CarCfg148/1 -> CCMSysServ_Runnable_sys/137(CarCfg_CarCfg148_read)
  CarCfg_CarCfg149/1 -> CCMSysServ_Runnable_sys/138(CarCfg_CarCfg149_read)
  CarCfg_CarCfg150/1 -> CCMSysServ_Runnable_sys/139(CarCfg_CarCfg150_read)
  CarCfg_CarCfg151/1 -> CCMSysServ_Runnable_sys/140(CarCfg_CarCfg151_read)
  CarCfg_CarCfg152/1 -> CCMSysServ_Runnable_sys/141(CarCfg_CarCfg152_read)
  CarCfg_CarCfg153/1 -> CCMSysServ_Runnable_sys/142(CarCfg_CarCfg153_read)
  CarCfg_CarCfg154/1 -> CCMSysServ_Runnable_sys/143(CarCfg_CarCfg154_read)
  CarCfg_CarCfg155/1 -> CCMSysServ_Runnable_sys/144(CarCfg_CarCfg155_read)
  CarCfg_CarCfg156/1 -> CCMSysServ_Runnable_sys/145(CarCfg_CarCfg156_read)
  CarCfg_CarCfg157/1 -> CCMSysServ_Runnable_sys/146(CarCfg_CarCfg157_read)
  CarCfg_CarCfg158/1 -> CCMSysServ_Runnable_sys/147(CarCfg_CarCfg158_read)
  CarCfg_CarCfg159/1 -> CCMSysServ_Runnable_sys/148(CarCfg_CarCfg159_read)
  CarCfg_CarCfg16/1 -> CCMSysServ_Runnable_sys/149(CarCfg_CarCfg16_read)
  CarCfg_CarCfg160/1 -> CCMSysServ_Runnable_sys/150(CarCfg_CarCfg160_read)
  CarCfg_CarCfg161/1 -> CCMSysServ_Runnable_sys/151(CarCfg_CarCfg161_read)
  CarCfg_CarCfg162/1 -> CCMSysServ_Runnable_sys/152(CarCfg_CarCfg162_read)
  CarCfg_CarCfg163/1 -> CCMSysServ_Runnable_sys/153(CarCfg_CarCfg163_read)
  CarCfg_CarCfg164/1 -> CCMSysServ_Runnable_sys/154(CarCfg_CarCfg164_read)
  CarCfg_CarCfg165/1 -> CCMSysServ_Runnable_sys/155(CarCfg_CarCfg165_read)
  CarCfg_CarCfg166/1 -> CCMSysServ_Runnable_sys/156(CarCfg_CarCfg166_read)
  CarCfg_CarCfg167/1 -> CCMSysServ_Runnable_sys/157(CarCfg_CarCfg167_read)
  CarCfg_CarCfg168/1 -> CCMSysServ_Runnable_sys/158(CarCfg_CarCfg168_read)
  CarCfg_CarCfg169/1 -> CCMSysServ_Runnable_sys/159(CarCfg_CarCfg169_read)
  CarCfg_CarCfg17/1 -> CCMSysServ_Runnable_sys/160(CarCfg_CarCfg17_read)
  CarCfg_CarCfg170/1 -> CCMSysServ_Runnable_sys/161(CarCfg_CarCfg170_read)
  CarCfg_CarCfg171/1 -> CCMSysServ_Runnable_sys/162(CarCfg_CarCfg171_read)
  CarCfg_CarCfg172/1 -> CCMSysServ_Runnable_sys/163(CarCfg_CarCfg172_read)
  CarCfg_CarCfg173/1 -> CCMSysServ_Runnable_sys/164(CarCfg_CarCfg173_read)
  CarCfg_CarCfg174/1 -> CCMSysServ_Runnable_sys/165(CarCfg_CarCfg174_read)
  CarCfg_CarCfg175/1 -> CCMSysServ_Runnable_sys/166(CarCfg_CarCfg175_read)
  CarCfg_CarCfg176/1 -> CCMSysServ_Runnable_sys/167(CarCfg_CarCfg176_read)
  CarCfg_CarCfg177/1 -> CCMSysServ_Runnable_sys/168(CarCfg_CarCfg177_read)
  CarCfg_CarCfg178/1 -> CCMSysServ_Runnable_sys/169(CarCfg_CarCfg178_read)
  CarCfg_CarCfg179/1 -> CCMSysServ_Runnable_sys/170(CarCfg_CarCfg179_read)
  CarCfg_CarCfg18/1 -> CCMSysServ_Runnable_sys/171(CarCfg_CarCfg18_read)
  CarCfg_CarCfg180/1 -> CCMSysServ_Runnable_sys/172(CarCfg_CarCfg180_read)
  CarCfg_CarCfg181/1 -> CCMSysServ_Runnable_sys/173(CarCfg_CarCfg181_read)
  CarCfg_CarCfg182/1 -> CCMSysServ_Runnable_sys/174(CarCfg_CarCfg182_read)
  CarCfg_CarCfg183/1 -> CCMSysServ_Runnable_sys/175(CarCfg_CarCfg183_read)
  CarCfg_CarCfg184/1 -> CCMSysServ_Runnable_sys/176(CarCfg_CarCfg184_read)
  CarCfg_CarCfg185/1 -> CCMSysServ_Runnable_sys/177(CarCfg_CarCfg185_read)
  CarCfg_CarCfg186/1 -> CCMSysServ_Runnable_sys/178(CarCfg_CarCfg186_read)
  CarCfg_CarCfg187/1 -> CCMSysServ_Runnable_sys/179(CarCfg_CarCfg187_read)
  CarCfg_CarCfg188/1 -> CCMSysServ_Runnable_sys/180(CarCfg_CarCfg188_read)
  CarCfg_CarCfg189/1 -> CCMSysServ_Runnable_sys/181(CarCfg_CarCfg189_read)
  CarCfg_CarCfg19/1 -> CCMSysServ_Runnable_sys/182(CarCfg_CarCfg19_read)
  CarCfg_CarCfg190/1 -> CCMSysServ_Runnable_sys/183(CarCfg_CarCfg190_read)
  CarCfg_CarCfg191/1 -> CCMSysServ_Runnable_sys/184(CarCfg_CarCfg191_read)
  CarCfg_CarCfg192/1 -> CCMSysServ_Runnable_sys/185(CarCfg_CarCfg192_read)
  CarCfg_CarCfg193/1 -> CCMSysServ_Runnable_sys/186(CarCfg_CarCfg193_read)
  CarCfg_CarCfg194/1 -> CCMSysServ_Runnable_sys/187(CarCfg_CarCfg194_read)
  CarCfg_CarCfg195/1 -> CCMSysServ_Runnable_sys/188(CarCfg_CarCfg195_read)
  CarCfg_CarCfg196/1 -> CCMSysServ_Runnable_sys/189(CarCfg_CarCfg196_read)
  CarCfg_CarCfg197/1 -> CCMSysServ_Runnable_sys/190(CarCfg_CarCfg197_read)
  CarCfg_CarCfg198/1 -> CCMSysServ_Runnable_sys/191(CarCfg_CarCfg198_read)
  CarCfg_CarCfg199/1 -> CCMSysServ_Runnable_sys/192(CarCfg_CarCfg199_read)
  CarCfg_CarCfg2/1 -> CCMSysServ_Runnable_sys/193(CarCfg_CarCfg2_read)
  CarCfg_CarCfg20/1 -> CCMSysServ_Runnable_sys/194(CarCfg_CarCfg20_read)
  CarCfg_CarCfg200/1 -> CCMSysServ_Runnable_sys/195(CarCfg_CarCfg200_read)
  CarCfg_CarCfg201/1 -> CCMSysServ_Runnable_sys/196(CarCfg_CarCfg201_read)
  CarCfg_CarCfg202/1 -> CCMSysServ_Runnable_sys/197(CarCfg_CarCfg202_read)
  CarCfg_CarCfg203/1 -> CCMSysServ_Runnable_sys/198(CarCfg_CarCfg203_read)
  CarCfg_CarCfg204/1 -> CCMSysServ_Runnable_sys/199(CarCfg_CarCfg204_read)
  CarCfg_CarCfg205/1 -> CCMSysServ_Runnable_sys/200(CarCfg_CarCfg205_read)
  CarCfg_CarCfg206/1 -> CCMSysServ_Runnable_sys/201(CarCfg_CarCfg206_read)
  CarCfg_CarCfg207/1 -> CCMSysServ_Runnable_sys/202(CarCfg_CarCfg207_read)
  CarCfg_CarCfg208/1 -> CCMSysServ_Runnable_sys/203(CarCfg_CarCfg208_read)
  CarCfg_CarCfg209/1 -> CCMSysServ_Runnable_sys/204(CarCfg_CarCfg209_read)
  CarCfg_CarCfg210/1 -> CCMSysServ_Runnable_sys/205(CarCfg_CarCfg210_read)
  CarCfg_CarCfg211/1 -> CCMSysServ_Runnable_sys/206(CarCfg_CarCfg211_read)
  CarCfg_CarCfg212/1 -> CCMSysServ_Runnable_sys/207(CarCfg_CarCfg212_read)
  CarCfg_CarCfg213/1 -> CCMSysServ_Runnable_sys/208(CarCfg_CarCfg213_read)
  CarCfg_CarCfg214/1 -> CCMSysServ_Runnable_sys/209(CarCfg_CarCfg214_read)
  CarCfg_CarCfg215/1 -> CCMSysServ_Runnable_sys/210(CarCfg_CarCfg215_read)
  CarCfg_CarCfg216/1 -> CCMSysServ_Runnable_sys/211(CarCfg_CarCfg216_read)
  CarCfg_CarCfg217/1 -> CCMSysServ_Runnable_sys/212(CarCfg_CarCfg217_read)
  CarCfg_CarCfg218/1 -> CCMSysServ_Runnable_sys/213(CarCfg_CarCfg218_read)
  CarCfg_CarCfg219/1 -> CCMSysServ_Runnable_sys/214(CarCfg_CarCfg219_read)
  CarCfg_CarCfg22/1 -> CCMSysServ_Runnable_sys/215(CarCfg_CarCfg22_read)
  CarCfg_CarCfg220/1 -> CCMSysServ_Runnable_sys/216(CarCfg_CarCfg220_read)
  CarCfg_CarCfg221/1 -> CCMSysServ_Runnable_sys/217(CarCfg_CarCfg221_read)
  CarCfg_CarCfg222/1 -> CCMSysServ_Runnable_sys/218(CarCfg_CarCfg222_read)
  CarCfg_CarCfg223/1 -> CCMSysServ_Runnable_sys/219(CarCfg_CarCfg223_read)
  CarCfg_CarCfg224/1 -> CCMSysServ_Runnable_sys/220(CarCfg_CarCfg224_read)
  CarCfg_CarCfg225/1 -> CCMSysServ_Runnable_sys/221(CarCfg_CarCfg225_read)
  CarCfg_CarCfg226/1 -> CCMSysServ_Runnable_sys/222(CarCfg_CarCfg226_read)
  CarCfg_CarCfg227/1 -> CCMSysServ_Runnable_sys/223(CarCfg_CarCfg227_read)
  CarCfg_CarCfg228/1 -> CCMSysServ_Runnable_sys/224(CarCfg_CarCfg228_read)
  CarCfg_CarCfg229/1 -> CCMSysServ_Runnable_sys/225(CarCfg_CarCfg229_read)
  CarCfg_CarCfg23/1 -> CCMSysServ_Runnable_sys/226(CarCfg_CarCfg23_read)
  CarCfg_CarCfg230/1 -> CCMSysServ_Runnable_sys/227(CarCfg_CarCfg230_read)
  CarCfg_CarCfg231/1 -> CCMSysServ_Runnable_sys/228(CarCfg_CarCfg231_read)
  CarCfg_CarCfg232/1 -> CCMSysServ_Runnable_sys/229(CarCfg_CarCfg232_read)
  CarCfg_CarCfg235/1 -> CCMSysServ_Runnable_sys/230(CarCfg_CarCfg235_read)
  CarCfg_CarCfg236/1 -> CCMSysServ_Runnable_sys/231(CarCfg_CarCfg236_read)
  CarCfg_CarCfg237/1 -> CCMSysServ_Runnable_sys/232(CarCfg_CarCfg237_read)
  CarCfg_CarCfg238/1 -> CCMSysServ_Runnable_sys/233(CarCfg_CarCfg238_read)
  CarCfg_CarCfg239/1 -> CCMSysServ_Runnable_sys/234(CarCfg_CarCfg239_read)
  CarCfg_CarCfg24/1 -> CCMSysServ_Runnable_sys/235(CarCfg_CarCfg24_read)
  CarCfg_CarCfg240/1 -> CCMSysServ_Runnable_sys/236(CarCfg_CarCfg240_read)
  CarCfg_CarCfg241/1 -> CCMSysServ_Runnable_sys/237(CarCfg_CarCfg241_read)
  CarCfg_CarCfg242/1 -> CCMSysServ_Runnable_sys/238(CarCfg_CarCfg242_read)
  CarCfg_CarCfg243/1 -> CCMSysServ_Runnable_sys/239(CarCfg_CarCfg243_read)
  CarCfg_CarCfg244/1 -> CCMSysServ_Runnable_sys/240(CarCfg_CarCfg244_read)
  CarCfg_CarCfg245/1 -> CCMSysServ_Runnable_sys/241(CarCfg_CarCfg245_read)
  CarCfg_CarCfg246/1 -> CCMSysServ_Runnable_sys/242(CarCfg_CarCfg246_read)
  CarCfg_CarCfg247/1 -> CCMSysServ_Runnable_sys/243(CarCfg_CarCfg247_read)
  CarCfg_CarCfg248/1 -> CCMSysServ_Runnable_sys/244(CarCfg_CarCfg248_read)
  CarCfg_CarCfg249/1 -> CCMSysServ_Runnable_sys/245(CarCfg_CarCfg249_read)
  CarCfg_CarCfg25/1 -> CCMSysServ_Runnable_sys/246(CarCfg_CarCfg25_read)